simulink model "ofdm_txrx_supermimo"  (Simulink 7.1)
settings: Solver=VariableStepDiscrete  SolverMode=SingleTasking  StartTime=0.0  StopTime=simOnly_simLength  RelTol=1e-3
PostLoadFcn:
  | ofdm_rx_supermimo_init
InitFcn:
  | ofdm_rx_supermimo_init
StartFcn:
  | ofdm_rx_supermimo_init
values from neighbouring files (symbols this model uses):
  Action0_Config = 0* 31*2^18 + 0*2^24 + PHY_AUTORESPONSE_REQ_GOODHDR + PHY_AUTORESPONSE_REQ_GOODPKT + PHY_AUTORESPONSE_REQ_FLAGA    [ofdm_tx_supermimo_init.m]
  Action1_Config = 0* 62*2^18 + PHY_AUTORESPONSE_REQ_GOODHDR + PHY_AUTORESPONSE_REQ_GOODPKT    [ofdm_tx_supermimo_init.m]
  Action2_Config = 0    [ofdm_tx_supermimo_init.m]
  Action3_Config = 0    [ofdm_tx_supermimo_init.m]
  Action4_Config = 0    [ofdm_tx_supermimo_init.m]
  Action5_Config = 0    [ofdm_tx_supermimo_init.m]
  ActionID_AFTransmit = 31    [ofdm_tx_supermimo_init.m]
  ActionID_Disabled = 0    [ofdm_tx_supermimo_init.m]
  ActionID_SetFlagA = 62    [ofdm_tx_supermimo_init.m]
  ActionID_SetFlagB = 61    [ofdm_tx_supermimo_init.m]
  BER_RAM_init_size = 2048    [ofdm_tx_supermimo_init.m]
  CPLength = 16    [ofdm_tx_supermimo_init.m]
  longCorr_coef_i = [-4*real(LTS) 0]    [ofdm_rx_supermimo_init.m]
  longCorr_coef_q = [4*imag(LTS) 0]    [ofdm_rx_supermimo_init.m]
  Match0_Config = 0    [ofdm_tx_supermimo_init.m]
  Match1_Config = 0    [ofdm_tx_supermimo_init.m]
  Match2_Config = 0    [ofdm_tx_supermimo_init.m]
  Match3_Config = 0    [ofdm_tx_supermimo_init.m]
  Match4_Config = 0    [ofdm_tx_supermimo_init.m]
  Match5_Config = 0    [ofdm_tx_supermimo_init.m]
  Match6_Config = 0    [ofdm_tx_supermimo_init.m]
  Match7_Config = 0    [ofdm_tx_supermimo_init.m]
  max_CP_length = 16    [ofdm_tx_supermimo_init.m]
  max_num_baseRateSymbols = 31    [ofdm_tx_supermimo_init.m]
  max_num_subcarriers = 64    [ofdm_tx_supermimo_init.m]
  max_num_trainingSymbols = 15    [ofdm_tx_supermimo_init.m]
  max_numBytes = 16384    [ofdm_tx_supermimo_init.m]
  max_OFDM_symbols = 511    [ofdm_tx_supermimo_init.m]
  modConstellation_qam16 = .75*[1 3 -1 -3]./3    [ofdm_tx_supermimo_init.m]
  modConstellation_qam64 = 0.875*[3 1 5 7 -3 -1 -5 -7]./7    [ofdm_tx_supermimo_init.m]
  modConstellation_qpsk = [1 -1]./sqrt(2)    [ofdm_tx_supermimo_init.m]
  numBaseRateSymbols = ceil(numHeaderBytes / numBytes_BaseRateOFDMSymbol)    [ofdm_tx_supermimo_init.m]
  numSubcarriers = 64    [ofdm_tx_supermimo_init.m]
  numTrainingSymbols = 2    [ofdm_tx_supermimo_init.m]
  pilot_indicies = 7 + ( (64-21) * 2^8) + ( (64-7) * 2^16) + (21 * 2^24)    (= 356068103)    [ofdm_tx_supermimo_init.m]
  pilotValues = hex2dec('A57D') + (2^16 * hex2dec('5A82'))    [ofdm_tx_supermimo_init.m]
  pkt_crossCorr_thresh = 0.7    [ofdm_rx_supermimo_init.m]
  pkt_energy_thresh = 0    [ofdm_rx_supermimo_init.m]
  pktByteNums = numHeaderBytes +  				byteIndex_numPayloadBytes(1)*2^8 +  				byteIndex_numPayloadBytes(2)*2^16 +  				byteIndex_simpleDynModMasks * 2^24    [ofdm_rx_supermimo_init.m]
  PktDet_Delay = 58+32 +      Symbol_Timing_Offset*2^7 +      0*2^16 +      (117* 2^24)    (= 1962935642)    [ofdm_rx_supermimo_init.m]
  pktTiming_count_payloadStart = pktTiming_count_lsStart + pktTiming_ls_num*pktTiming_ls_length    [ofdm_rx_supermimo_init.m]
  preamble = preamble_scale*[shortsyms_10 longsyms_2]    [ofdm_tx_supermimo_init.m]
  preamble_ROM = preamble_I_ROM + preamble_Q_ROM*2^16    [ofdm_tx_supermimo_init.m]
  RAM_init_size = max_numBytes    (= 16384)    [ofdm_tx_supermimo_init.m]
  reg_AF_Tx_Blanking = (320+80) + ( (320+80+79)*2^16)    (= 31392144)    [ofdm_rx_supermimo_init.m]
  reg_AF_Tx_Scaling = 2944    [ofdm_rx_supermimo_init.m]
  reg_Interrupt_PktBuf_Control = reg_InterruptControl +  	1 * 2^16 +  	0 * 2^24    (= 65656)    [ofdm_rx_supermimo_init.m]
  reg_PilotCalcParams = (4 * 2^16) + (minPilotChanMag)    (= 262184.96)    [ofdm_rx_supermimo_init.m]
  regRx_chanEst_minMag = ((0.5)*2^15) + ((0.5 * 2^15)*2^16)    (= 1073758208)    [ofdm_rx_supermimo_init.m]
  regRx_coarseCalcCorrection = round((1.3e-3 - 1.2982e-3)*2^32)    [ofdm_rx_supermimo_init.m]
  regRx_pilotCalcCorrection = round(1.00115 * 2^31)    [ofdm_rx_supermimo_init.m]
  regRx_preCFOoptions = 0 * 2^0 +  	1 * 2^1 +  	0    (= 2)    [ofdm_rx_supermimo_init.m]
  rx_controlBits = 1 * 2  		 + 1 * 4  		 + 0 * 8  		 + 1 * 16 * rx_SISO_Mode  		 + 1 * 32  		 + 1 * 64  		 + 1 * 128  		 + 1 * 256  		 + 1 * 512  		 + 1 * 1024  		 + 1 * 4096  		 + 0 * 16384  		 + 0 * 32768  		 + 1 * 65536  		 + 0 * 131072  		 + 0 * 262144  		 + 1 * 524288  		 + 1 * 1048576 * tx_Alamouti_Mode  		 + 0 * 2097152           + 1 * 33554432  		 + 0 * 4194304  		 + 0 * 8388608  		 + 0 * 16777216  		 + 1 *  ...(+49 chars)    [ofdm_rx_supermimo_init.m]
  Rx_PktDet_LongCorr_Params = LongCorrCounterLoad + 0*2^8 + 0*2^16 + 255*2^24    (= 4278190325)    [ofdm_rx_supermimo_init.m]
  Rx_PktDet_LongCorrThresholds = 8000    [ofdm_rx_supermimo_init.m]
  rx_postEq_scaling = round(rxScaling*2^11) * (1 + 2^16)    [ofdm_rx_supermimo_init.m]
  RxDataScrambling_Seq = [40 198 78 63 82 173 102 245 48 111 172 115 147 230 216 93 72 65 62 2 205 242 122 90 128 83 105 97 73 10 5 252]    [ofdm_tx_supermimo_init.m]
  RxReg_FixedPktLen = 0    [ofdm_rx_supermimo_init.m]
  shortSymbol_time = shortSymbol_time(1:16).'    [ofdm_tx_supermimo_init.m]
  simOnly_numSamples = length(preamble)+( (numSubcarriers+CPLength)*(numTrainingSymbols + numBaseRateSymbols + numFullRateSymbols/2) )    [ofdm_tx_supermimo_init.m]
  train = [train train]    [ofdm_tx_supermimo_init.m]
  tx_controlBits = (antB_preambleShift * 2^4) + (2^1 * tx_Alamouti_Mode) +  	(2^0 * tx_SISO_Mode) + 4*0 + 8*1 + 0*256 + 512*0 + 1024*0 +  	0*hex2dec('1000') + 0*hex2dec('2000') + 0*hex2dec('4000') +  	1*hex2dec('8000') + 1*hex2dec('10000') + hex2dec('20000') +  	16*hex2dec('100000')    [ofdm_tx_supermimo_init.m]
  tx_delays = (50*2^0) + (0*2^8) + (30*2^12)    (= 122930)    [ofdm_tx_supermimo_init.m]
  tx_scaling = (3072) + (12288 * 2^16)    (= 805309440)    [ofdm_tx_supermimo_init.m]
  TxDataScrambling_Seq = [40 198 78 63 82 173 102 245 48 111 172 115 147 230 216 93 72 65 62 2 205 242 122 90 128 83 105 97 73 10 5 252]    [ofdm_tx_supermimo_init.m]
  txfilt_firpm = 0.95*txfilt_firpm./(max(txfilt_firpm))    [ofdm_tx_supermimo_init.m]
  txfilt_intfilt = [0 intfilt(4,4,.8)]    [ofdm_tx_supermimo_init.m]
  txReg_symbolCounts = (2^8 *numBaseRateSymbols ) + numTrainingSymbols    [ofdm_tx_supermimo_init.m]
  TxRx_FFTScaling = (3 * 2^0) +  	(2 * 2^2) +  	(1 * 2^4) +  	(1 * 2^6) +  	(1 * 2^8) +  	(0 * 2^10)    (= 347)    [ofdm_tx_supermimo_init.m]
undefined symbols (not defined in the file or in its folder): AntA, AntB, BaseRate, Down, gcbh, numBuffs, numBytes, Up, xlBlockLoadCallback, xlGetSimulinkPeriod, xlipgetpartsetting
libraries: xbsIndex_r4 x6035, simulink x4, dspsigops x4, commchan3 x2, dspsrcs4 x2, commrflib2 x2
(part 19 of 61)

== system ofdm_txrx_supermimo/OFDM Rx MIMO/Equalizer & Packetizer/Equalizer/Determinant ==
  note: In SISO and Multiplexing mode, this block
      | calculates the determinant of the 2x2 matrix:
      | det([a b; c d]) = ad - bc
      | where a,b,c,d are all complex
      | In Alamouti mode, it calculates the sum of
      | the magnitude of the two channel coefficients
      | |a|^2 + |b|^2
  Re[a] : Inport  OutDataType=sfix(16)  OutScaling=2^0
  Im[a] : Inport  Port=2  OutDataType=sfix(16)  OutScaling=2^0
  Re[d] : Inport  Port=3  OutDataType=sfix(16)  OutScaling=2^0
  Im[d] : Inport  Port=4  OutDataType=sfix(16)  OutScaling=2^0
  Re[b] : Inport  Port=5  OutDataType=sfix(16)  OutScaling=2^0
  Im[b] : Inport  Port=6  OutDataType=sfix(16)  OutScaling=2^0
  Re[c] : Inport  Port=7  OutDataType=sfix(16)  OutScaling=2^0
  Im[c] : Inport  Port=8  OutDataType=sfix(16)  OutScaling=2^0
  Add1 : xbsIndex_r4/AddSub  mode=Addition or Subtraction  use_carryin=off  use_carryout=off  en=off  latency=0  precision=User Defined  arith_type="Signed  (2's comp)"  n_bits=22  bin_pt=17  quantization=Truncate  overflow=Flag as error  dbl_ovrd=off  use_behavioral_HDL=on  pipelined=off  use_rpm=on  hw_selection=Fabric  block_type=addsub
      settings: xl_area=[9 0 0 16 0 0 0]  block_version=VER_STRING_GOES_HERE
  Add2 : xbsIndex_r4/AddSub  mode=Addition or Subtraction  use_carryin=off  use_carryout=off  en=off  latency=0  precision=User Defined  arith_type="Signed  (2's comp)"  n_bits=22  bin_pt=17  quantization=Truncate  overflow=Flag as error  dbl_ovrd=off  use_behavioral_HDL=on  pipelined=off  use_rpm=on  hw_selection=Fabric  block_type=addsub
      settings: xl_area=[9 0 0 16 0 0 0]  block_version=VER_STRING_GOES_HERE
  ComplexMult_x1 : Subsystem
  ComplexMult_x2 : Subsystem
  Inverter : xbsIndex_r4/Inverter  en=off  latency=0  dbl_ovrd=off  block_type=inv
  Re : Outport  OutDataType=sfix(16)  OutScaling=2^0
  Im : Outport  Port=2  OutDataType=sfix(16)  OutScaling=2^0
  wires:
  ComplexMult_x2/1(Re) -> Add2/1
  ComplexMult_x1/1(Re) -> Add2/2
  ComplexMult_x2/2(Im) -> Add1/1
  ComplexMult_x1/2(Im) -> Add1/2
  Re[a]/1 -> ComplexMult_x2/1(Re[A])
  Im[a]/1 -> ComplexMult_x2/2(Im[A])
  Add2/1 -> Re/1
  Re[b]/1 -> ComplexMult_x1/1(Re[A])
  Add1/1 -> Im/1
  Im[b]/1 -> ComplexMult_x1/2(Im[A])
  [regRx_AlamoutiMode] -> Inverter/1
  Inverter/1 -> Add2/3, Add1/3
  Re[c]/1 -> ComplexMult_x1/3(Re[B])
  Im[c]/1 -> ComplexMult_x1/4(Im[B])
  Re[d]/1 -> ComplexMult_x2/3(Re[B])
  Im[d]/1 -> ComplexMult_x2/4(Im[B])

== system ofdm_txrx_supermimo/OFDM Rx MIMO/Equalizer & Packetizer/Equalizer/Phase Corr ==
  note: For SISO/Multiplexing, update the phase correction
      | value for each new symbol.
      | For Alamouti, update it every other symbol
      | (since in Alamouti mode the sym index increments
      | twice for each pair of coincident symbols).
  Vin : Inport  OutDataType=sfix(16)  OutScaling=2^0
  xk_ind : Inport  Port=2  OutDataType=sfix(16)  OutScaling=2^0
  Phases I : Inport  Port=3  OutDataType=sfix(16)  OutScaling=2^0
  Phases Q : Inport  Port=4  OutDataType=sfix(16)  OutScaling=2^0
  YI_A : Inport  Port=5
  YQ_A : Inport  Port=6
  YI_B : Inport  Port=7
  YQ_B : Inport  Port=8
  Bus Selector1 : BusSelector  OutputSignals=signal1,signal2
  Bus Selector2 : BusSelector  OutputSignals=signal1,signal2
  ComplexMult_x3 : Subsystem
  ComplexMult_x4 : Subsystem
  Phase Corr : Scope  NumInputPorts=7
      settings: TimeRange=392.7801724137931  YMin=-0.5~-0.25~-1~-1~0~0~0  YMax=0.5~0.15~1~1~1~3500~1  SaveName=ScopeData21
  done{1..7} (7 alike) : xbsIndex_r4/Gateway Out  hdl_port=off  timing_constraint=None  locs_specified=off  LOCs={}  block_type=gatewayout
      settings: infoedit="Gateway out block.  Converts Xilinx fixed point inputs into ouputs of type Simulink integer, double, or fixed point.<P><P>Hardware notes:  In hardware these blocks become top level output ports or are discarded, depending on how they are configured."  xl_area=[0 0 0 0 1 0 0]  block_version=VER_STRING_GOES_HERE
  " YI_A" : Outport
  " YQ_A" : Outport  Port=2
  " YI_B" : Outport  Port=3
  " YQ_B" : Outport  Port=4
  wires:
  ComplexMult_x4/1(Re) -> " YI_B"/1
  ComplexMult_x4/2(Im) -> " YQ_B"/1
  YI_B/1 -> ComplexMult_x4/1(Re[A]), done7/1
  YQ_B/1 -> ComplexMult_x4/2(Im[A])
  YI_A/1 -> ComplexMult_x3/1(Re[A]), done6/1
  YQ_A/1 -> ComplexMult_x3/2(Im[A])
  ComplexMult_x3/1(Re) -> " YI_A"/1
  ComplexMult_x3/2(Im) -> " YQ_A"/1
  Phases I/1 -> Bus Selector1/1
  Bus Selector1/1 -> ComplexMult_x3/3(Re[B]), done5/1  "<signal1>"
  Bus Selector1/2 -> ComplexMult_x4/3(Re[B]), done2/1  "<signal2>"
  Phases Q/1 -> Bus Selector2/1
  Bus Selector2/1 -> ComplexMult_x3/4(Im[B]), done1/1  "<signal1>"
  Bus Selector2/2 -> ComplexMult_x4/4(Im[B]), done3/1  "<signal2>"
  done4/1 -> Phase Corr/1
  done5/1 -> Phase Corr/2
  xk_ind/1 -> done4/1
  done1/1 -> Phase Corr/3
  done2/1 -> Phase Corr/4
  done3/1 -> Phase Corr/5
  done6/1 -> Phase Corr/6
  done7/1 -> Phase Corr/7

== system ofdm_txrx_supermimo/OFDM Rx MIMO/Equalizer & Packetizer/Equalizer/YA*[HBB,-HAB] ==
  Re[y] : Inport  OutDataType=sfix(16)  OutScaling=2^0
  Im[y] : Inport  Port=2  OutDataType=sfix(16)  OutScaling=2^0
  Re[h1] : Inport  Port=3  OutDataType=sfix(16)  OutScaling=2^0
  Im[h1] : Inport  Port=4  OutDataType=sfix(16)  OutScaling=2^0
  Re[h2] : Inport  Port=5  OutDataType=sfix(16)  OutScaling=2^0
  Im[h2] : Inport  Port=6  OutDataType=sfix(16)  OutScaling=2^0
  Slot : Inport  Port=7  OutDataType=sfix(16)  OutScaling=2^0
  ComplexMult_x1 : Subsystem
  Concat : xbsIndex_r4/Concat  num_inputs=2  dbl_ovrd=off  block_type=concat
      settings: block_version=10.1.3
  Mux1 : xbsIndex_r4/Mux  inputs=4  en=off  latency=0  precision=Full  arith_type="Signed  (2's comp)"  n_bits=16  bin_pt=13  quantization=Truncate  overflow=Wrap  dbl_ovrd=off  block_type=mux
      settings: xl_area=[14 0 0 28 0 0 0]  block_version=VER_STRING_GOES_HERE
  Mux2 : xbsIndex_r4/Mux  inputs=4  en=off  latency=0  precision=Full  arith_type="Signed  (2's comp)"  n_bits=16  bin_pt=13  quantization=Truncate  overflow=Wrap  dbl_ovrd=off  block_type=mux
      settings: xl_area=[14 0 0 28 0 0 0]  block_version=VER_STRING_GOES_HERE
  Negate1 : xbsIndex_r4/Negate  precision=User Defined  arith_type="Signed  (2's comp)"  n_bits=18  bin_pt=16  quantization=Truncate  overflow=Flag as error  en=off  latency=0  dbl_ovrd=off  block_type=negate
      settings: xl_area=[7 0 0 14 0 0 0]  block_version=VER_STRING_GOES_HERE
  Negate2 : xbsIndex_r4/Negate  precision=User Defined  arith_type="Signed  (2's comp)"  n_bits=18  bin_pt=16  quantization=Truncate  overflow=Flag as error  en=off  latency=0  dbl_ovrd=off  block_type=negate
      settings: xl_area=[7 0 0 14 0 0 0]  block_version=VER_STRING_GOES_HERE
  Re : Outport  OutDataType=sfix(16)  OutScaling=2^0
  Im : Outport  Port=2  OutDataType=sfix(16)  OutScaling=2^0
  wires:
  Re[y]/1 -> ComplexMult_x1/1(Re[A])
  ComplexMult_x1/1(Re) -> Re/1
  Im[y]/1 -> ComplexMult_x1/2(Im[A])
  Re[h1]/1 -> Mux2/4, Mux2/2
  ComplexMult_x1/2(Im) -> Im/1
  Re[h2]/1 -> Negate2/1, Mux2/5
  Im[h2]/1 -> Negate1/1, Mux1/5
  Negate2/1 -> Mux2/3
  [regRx_AlamoutiMode] -> Concat/1
  Slot/1 -> Concat/2
  Concat/1 -> Mux1/1, Mux2/1
  Im[h1]/1 -> Mux1/2, Mux1/4
  Mux1/1 -> ComplexMult_x1/4(Im[B])
  Mux2/1 -> ComplexMult_x1/3(Re[B])
  Negate1/1 -> Mux1/3

== system ofdm_txrx_supermimo/OFDM Rx MIMO/Equalizer & Packetizer/Equalizer/YB*[-HBA,HAA] ==
  Re[y] : Inport  OutDataType=sfix(16)  OutScaling=2^0
  Im[y] : Inport  Port=2  OutDataType=sfix(16)  OutScaling=2^0
  Re[h1] : Inport  Port=3  OutDataType=sfix(16)  OutScaling=2^0
  Im[h1] : Inport  Port=4  OutDataType=sfix(16)  OutScaling=2^0
  Re[h2] : Inport  Port=5  OutDataType=sfix(16)  OutScaling=2^0
  Im[h2] : Inport  Port=6  OutDataType=sfix(16)  OutScaling=2^0
  Slot : Inport  Port=7  OutDataType=sfix(16)  OutScaling=2^0
  ComplexMult_x2 : Subsystem
  Concat : xbsIndex_r4/Concat  num_inputs=2  dbl_ovrd=off  block_type=concat
      settings: block_version=10.1.3
  Mux1 : xbsIndex_r4/Mux  inputs=2  en=off  latency=0  precision=Full  arith_type="Signed  (2's comp)"  n_bits=16  bin_pt=13  quantization=Truncate  overflow=Wrap  dbl_ovrd=off  block_type=mux
      settings: xl_area=[14 0 0 28 0 0 0]  block_version=VER_STRING_GOES_HERE
  Mux2 : xbsIndex_r4/Mux  inputs=4  en=off  latency=0  precision=Full  arith_type="Signed  (2's comp)"  n_bits=16  bin_pt=13  quantization=Truncate  overflow=Wrap  dbl_ovrd=off  block_type=mux
      settings: xl_area=[14 0 0 28 0 0 0]  block_version=VER_STRING_GOES_HERE
  Mux3 : xbsIndex_r4/Mux  inputs=4  en=off  latency=0  precision=Full  arith_type="Signed  (2's comp)"  n_bits=16  bin_pt=13  quantization=Truncate  overflow=Wrap  dbl_ovrd=off  block_type=mux
      settings: xl_area=[14 0 0 28 0 0 0]  block_version=VER_STRING_GOES_HERE
  Negate{1..5} (5 alike) : xbsIndex_r4/Negate  precision=User Defined  arith_type="Signed  (2's comp)"  n_bits=18  bin_pt=16  quantization=Truncate  overflow=Flag as error  en=off  latency=0  dbl_ovrd=off  block_type=negate
      settings: xl_area=[7 0 0 14 0 0 0]  block_version=VER_STRING_GOES_HERE
  Re : Outport  OutDataType=sfix(16)  OutScaling=2^0
  Im : Outport  Port=2  OutDataType=sfix(16)  OutScaling=2^0
  wires:
  Im[h1]/1 -> Negate2/1, Mux2/4
  ComplexMult_x2/2(Im) -> Im/1
  Re[h1]/1 -> Negate1/1, Mux3/4
  ComplexMult_x2/1(Re) -> Re/1
  [regRx_AlamoutiMode] -> Concat/1, Mux1/1
  Slot/1 -> Concat/2
  Concat/1 -> Mux3/1, Mux2/1
  Negate1/1 -> Mux3/2
  Negate2/1 -> Mux2/2
  Re[h2]/1 -> Mux3/3, Negate3/1
  Im[h2]/1 -> Mux2/3, Negate4/1
  Negate3/1 -> Mux3/5
  Negate4/1 -> Mux2/5
  Re[y]/1 -> ComplexMult_x2/1(Re[A])
  Mux1/1 -> ComplexMult_x2/2(Im[A])
  Im[y]/1 -> Mux1/2, Negate5/1
  Negate5/1 -> Mux1/3
  Mux3/1 -> ComplexMult_x2/3(Re[B])
  Mux2/1 -> ComplexMult_x2/4(Im[B])

== system ofdm_txrx_supermimo/OFDM Rx MIMO/Equalizer & Packetizer/Packet_Constructor/   ==
  signal : Inport  OutDataType=sfix(16)  OutScaling=2^0
  Delay1 : xbsIndex_r4/Delay  en=off  latency=1  dbl_ovrd=off  reg_retiming=off  block_type=delay
      settings: infoedit=Hardware notes: A delay line is a chain, each link of which is an SRL16 followed by a flip-flop. If register retiming is enabled, the delay line is a chain of flip-flops.  xl_area=[1 1 0 0 0 0 0]  block_version=VER_STRING_GOES_HERE
  Inverter1 : xbsIndex_r4/Inverter  en=off  latency=0  dbl_ovrd=off  block_type=inv
      settings: xl_area=[0 0 0 0 0 0 0]  block_version=VER_STRING_GOES_HERE
  Logical1 : xbsIndex_r4/Logical  logical_function=AND  inputs=2  en=off  latency=0  precision=Full  arith_type=Unsigned  n_bits=8  bin_pt=2  align_bp=on  dbl_ovrd=off  block_type=logical
      settings: xl_area=[1 0 0 1 0 0 0]  block_version=VER_STRING_GOES_HERE
  pulse : Outport  OutDataType=sfix(16)  OutScaling=2^0
  wires:
  Inverter1/1 -> Delay1/1
  signal/1 -> Inverter1/1, Logical1/1
  Delay1/1 -> Logical1/2
  Logical1/1 -> pulse/1

== system ofdm_txrx_supermimo/OFDM Rx MIMO/Equalizer & Packetizer/Packet_Constructor/Barrel Shifter ==
  ShiftSel : Inport  OutDataType=sfix(16)  OutScaling=2^0
  D : Inport  Port=2  OutDataType=sfix(16)  OutScaling=2^0
  en : Inport  Port=3  OutDataType=sfix(16)  OutScaling=2^0
  AddSub1 : xbsIndex_r4/AddSub  mode=Addition  use_carryin=off  use_carryout=off  en=off  latency=0  precision=User Defined  arith_type=Unsigned  n_bits=15  bin_pt=0  quantization=Truncate  overflow=Wrap  dbl_ovrd=off  use_behavioral_HDL=on  pipelined=off  use_rpm=on  hw_selection=Fabric  block_type=addsub
      settings: xl_area=[8 0 0 15 0 0 0]  block_version=VER_STRING_GOES_HERE
  Assert2 : xbsIndex_r4/Assert  Orientation=down  assert_type=off  type_source=Explicitly  arith_type="Signed  (2's comp)"  n_bits=16  bin_pt=14  assert_rate=on  rate_source=Explicitly  period=2  output_port=on  block_type=assert
      settings: infoedit=Asserts a user-defined sample rate and/or type on a signal.<P><P>Hardware notes: In hardware this block costs nothing.  block_version=VER_STRING_GOES_HERE
  Data Buffer : xbsIndex_r4/Register  Orientation=left  init=0  rst=off  en=on  dbl_ovrd=off  block_type=register
      settings: xl_area=[8 15 0 0 0 0 0]  block_version=VER_STRING_GOES_HERE
  Delay2 : xbsIndex_r4/Delay  en=off  latency=1  dbl_ovrd=off  reg_retiming=off  block_type=delay
      settings: infoedit=Hardware notes: A delay line is a chain, each link of which is an SRL16 followed by a flip-flop. If register retiming is enabled, the delay line is a chain of flip-flops.  xl_area=[1 1 0 0 0 0 0]  block_version=VER_STRING_GOES_HERE
  Shift : xbsIndex_r4/Shift  shift_dir=Left  shift_bits=1  en=off  latency=0  precision=User Defined  arith_type=Unsigned  n_bits=15  bin_pt=0  quantization=Truncate  overflow=Wrap  dbl_ovrd=off  block_type=shift
      settings: xl_area=[0 0 0 0 0 0 0]  block_version=VER_STRING_GOES_HERE
  Shift1 : xbsIndex_r4/Shift  shift_dir=Left  shift_bits=2  en=off  latency=0  precision=User Defined  arith_type=Unsigned  n_bits=15  bin_pt=0  quantization=Truncate  overflow=Wrap  dbl_ovrd=off  block_type=shift
      settings: xl_area=[0 0 0 0 0 0 0]  block_version=VER_STRING_GOES_HERE
  Shift2 : xbsIndex_r4/Shift  shift_dir=Left  shift_bits=4  en=off  latency=0  precision=User Defined  arith_type=Unsigned  n_bits=15  bin_pt=0  quantization=Truncate  overflow=Wrap  dbl_ovrd=off  block_type=shift
      settings: xl_area=[0 0 0 0 0 0 0]  block_version=VER_STRING_GOES_HERE
  Shift3 : xbsIndex_r4/Shift  shift_dir=Left  shift_bits=6  en=off  latency=0  precision=User Defined  arith_type=Unsigned  n_bits=15  bin_pt=0  quantization=Truncate  overflow=Wrap  dbl_ovrd=off  block_type=shift
      settings: xl_area=[0 0 0 0 0 0 0]  block_version=VER_STRING_GOES_HERE
  Shift4 : xbsIndex_r4/Shift  shift_dir=Left  shift_bits=8  en=off  latency=0  precision=User Defined  arith_type=Unsigned  n_bits=15  bin_pt=0  quantization=Truncate  overflow=Wrap  dbl_ovrd=off  block_type=shift
      settings: xl_area=[0 0 0 0 0 0 0]  block_version=VER_STRING_GOES_HERE
  Shift5 : xbsIndex_r4/Shift  shift_dir=Left  shift_bits=3  en=off  latency=0  precision=User Defined  arith_type=Unsigned  n_bits=15  bin_pt=0  quantization=Truncate  overflow=Wrap  dbl_ovrd=off  block_type=shift
      settings: xl_area=[0 0 0 0 0 0 0]  block_version=VER_STRING_GOES_HERE
  Shift6 : xbsIndex_r4/Shift  shift_dir=Left  shift_bits=5  en=off  latency=0  precision=User Defined  arith_type=Unsigned  n_bits=15  bin_pt=0  quantization=Truncate  overflow=Wrap  dbl_ovrd=off  block_type=shift
      settings: xl_area=[0 0 0 0 0 0 0]  block_version=VER_STRING_GOES_HERE
  Shift7 : xbsIndex_r4/Shift  shift_dir=Left  shift_bits=7  en=off  latency=0  precision=User Defined  arith_type=Unsigned  n_bits=15  bin_pt=0  quantization=Truncate  overflow=Wrap  dbl_ovrd=off  block_type=shift
      settings: xl_area=[0 0 0 0 0 0 0]  block_version=VER_STRING_GOES_HERE
  shift selector : xbsIndex_r4/Mux  inputs=9  en=off  latency=0  precision=Full  arith_type="Signed  (2's comp)"  n_bits=8  bin_pt=2  quantization=Truncate  overflow=Wrap  dbl_ovrd=off  block_type=mux
      settings: xl_area=[68 0 0 135 0 0 0]  block_version=VER_STRING_GOES_HERE
  Q : Outport  OutDataType=sfix(16)  OutScaling=2^0
  wires:
  D/1 -> Delay2/1
  ShiftSel/1 -> shift selector/1
  Shift7/1 -> shift selector/9
  Shift6/1 -> shift selector/7
  Shift5/1 -> shift selector/5
  shift selector/1 -> AddSub1/2
  Shift4/1 -> shift selector/10
  Shift3/1 -> shift selector/8
  Shift2/1 -> shift selector/6
  Shift1/1 -> shift selector/4
  Shift/1 -> shift selector/3
  AddSub1/1 -> Assert2/1
  Data Buffer/1 -> Shift5/1, shift selector/2, Shift/1, Shift1/1, Shift2/1, Shift6/1, Shift3/1, Shift7/1, Shift4/1
  en/1 -> Data Buffer/2
  Delay2/1 -> AddSub1/1
  Assert2/1 -> Q/1, Data Buffer/1

== system ofdm_txrx_supermimo/OFDM Rx MIMO/Equalizer & Packetizer/Packet_Constructor/Data Buffer ==
  Payload_Addr : Inport  OutDataType=sfix(16)  OutScaling=2^0
  Payload_WE : Inport  Port=2  OutDataType=sfix(16)  OutScaling=2^0
  Payload_Data : Inport  Port=3  OutDataType=sfix(16)  OutScaling=2^0
  Rst : Inport  Port=4  OutDataType=sfix(16)  OutScaling=2^0
  PktDone : Inport  Port=5  OutDataType=sfix(16)  OutScaling=2^0
  AutoResponse Processing : Subsystem
  BER Calc : Subsystem
  Descrambling & Pkt Buffer : Subsystem
  Error Checking : Subsystem
  Header Decoding : Subsystem
  numBytes : Outport  OutDataType=sfix(16)  OutScaling=2^0
  modMask : Outport  Port=2  OutDataType=sfix(16)  OutScaling=2^0
  ResetRx : Outport  Port=3  OutDataType=sfix(16)  OutScaling=2^0
  wires:
  Payload_Addr/1 -> Descrambling & Pkt Buffer/1(Addr)
  Payload_Data/1 -> Descrambling & Pkt Buffer/2(Data)
  Payload_WE/1 -> Descrambling & Pkt Buffer/3(We)
  Rst/1 -> Error Checking/5(Rst), AutoResponse Processing/1(Rst), Header Decoding/4(Rst), BER Calc/4(PktStart)
  Descrambling & Pkt Buffer/1(" Addr") -> Header Decoding/1(RxByteAddr), Error Checking/2(ByteAddr), AutoResponse Processing/2(RxByteAddr), BER Calc/1(BER_addr)
  Descrambling & Pkt Buffer/2(" Data") -> Header Decoding/2(RxByte), Error Checking/3(ByteIn), AutoResponse Processing/3(RxByte), BER Calc/2(BER_data)
  Descrambling & Pkt Buffer/3(" Vout") -> Header Decoding/3(RxByteWE), Error Checking/4(Vin), AutoResponse Processing/4(RxByteWE), BER Calc/3(BER_val)
  Header Decoding/1(numBytes) -> Error Checking/1(NumBytes), BER Calc/5(numBytes), numBytes/1
  Header Decoding/2(modMask) -> modMask/1
  PktDone/1 -> AutoResponse Processing/5(PktDone)
  Error Checking/1(ResetRx) -> ResetRx/1

== system ofdm_txrx_supermimo/OFDM Rx MIMO/Equalizer & Packetizer/Packet_Constructor/EVM Calc ==
  Rx Bits : Inport
  Mod Sel : Inport  Port=2
  Eq I : Inport  Port=3
  Eq Q : Inport  Port=4
  Xk_ind : Inport  Port=5
  Sym_ind : Inport  Port=6
  Vin : Inport  Port=7
  Done : Inport  Port=8
  AddSub : xbsIndex_r4/AddSub  mode=Subtraction  use_carryin=off  use_carryout=off  en=off  latency=0  precision=User Defined  arith_type="Signed  (2's comp)"  n_bits=16  bin_pt=13  quantization=Truncate  overflow=Flag as error  dbl_ovrd=off  use_behavioral_HDL=off  pipelined=off  use_rpm=on  hw_selection=Fabric  block_type=addsub
      settings: block_version=10.1.3
  AddSub1 : xbsIndex_r4/AddSub  mode=Subtraction  use_carryin=off  use_carryout=off  en=off  latency=0  precision=User Defined  arith_type="Signed  (2's comp)"  n_bits=16  bin_pt=13  quantization=Truncate  overflow=Flag as error  dbl_ovrd=off  use_behavioral_HDL=off  pipelined=off  use_rpm=on  hw_selection=Fabric  block_type=addsub
      settings: block_version=10.1.3
  ComplexMag (Latency=2) : Subsystem
  Constant : xbsIndex_r4/Constant  arith_type=Unsigned  const=0  n_bits=1  bin_pt=0  explicit_period=off  period=1  equ=P=C  opselect=C  inp2=PCIN>>17  opr=+  inp1=P  carry=CIN  dbl_ovrd=off  block_type=constant
  Convert1 : xbsIndex_r4/Convert  arith_type="Signed  (2's comp)"  n_bits=14  bin_pt=13  quantization=Truncate  overflow=Saturate  latency=0  dbl_ovrd=off  pipeline=off  block_type=convert
      settings: xl_area=[0, 0, 0, 0, 0, 0, 0]  block_version=VER_STRING_GOES_HERE
  Delay1 : xbsIndex_r4/Delay  en=off  latency=2  dbl_ovrd=off  reg_retiming=off  block_type=delay
  Delay2 : xbsIndex_r4/Delay  en=off  latency=4  dbl_ovrd=off  reg_retiming=off  block_type=delay
  Delay3 : xbsIndex_r4/Delay  en=off  latency=2  dbl_ovrd=off  reg_retiming=off  block_type=delay
  Delay4 : xbsIndex_r4/Delay  en=off  latency=2  dbl_ovrd=off  reg_retiming=off  block_type=delay
  Delay5 : xbsIndex_r4/Delay  en=off  latency=4  dbl_ovrd=off  reg_retiming=off  block_type=delay
  Delay6 : xbsIndex_r4/Delay  en=off  latency=4  dbl_ovrd=off  reg_retiming=off  block_type=delay
  Delay7 : xbsIndex_r4/Delay  en=off  latency=1  dbl_ovrd=off  reg_retiming=off  block_type=delay
  Delay8 : xbsIndex_r4/Delay  en=off  latency=1  dbl_ovrd=off  reg_retiming=off  block_type=delay
  "EVM " : Scope  NumInputPorts=7
      settings: TimeRange=8000  YMin=0~0~-0.75~-0.75~0~0~0  YMax=70~12.5~0.75~0.75~1~1~1  SaveName=ScopeData78
  EVM per Subcarrier : Subsystem
  EVM per Symbol : Subsystem
  Inverter : xbsIndex_r4/Inverter  en=off  latency=0  dbl_ovrd=off  block_type=inv
  Logical : xbsIndex_r4/Logical  logical_function=OR  inputs=3  en=off  latency=0  precision=Full  arith_type=Unsigned  n_bits=16  bin_pt=0  align_bp=on  dbl_ovrd=off  block_type=logical
  Posedge6 : Subsystem
  Re-modulator : Subsystem
  Register2 : xbsIndex_r4/Register  init=0  rst=on  en=off  dbl_ovrd=off  block_type=register
  Relational : xbsIndex_r4/Relational  mode=a=b  en=off  latency=1  dbl_ovrd=off  block_type=relational
      settings: block_version=10.1.3
  Rx_debug_EVM : xbsIndex_r4/Gateway Out  hdl_port=on  timing_constraint=None  locs_specified=off  LOCs={}  block_type=gatewayout
      settings: infoedit="Gateway out block.  Converts Xilinx fixed point inputs into ouputs of type Simulink integer, double, or fixed point.<P><P>Hardware notes:  In hardware these blocks become top level output ports or are discarded, depending on how they are configured."  xl_area=[0 0 0 0 1 0 0]  block_version=VER_STRING_GOES_HERE
  SRLatch : Subsystem
  Up Sample : xbsIndex_r4/Up Sample  sample_ratio=2  copy_samples=on  dbl_ovrd=off  block_type=usamp
      settings: infoedit="Up samples input data.  Inserted values  can be zeros or copies of the most recent input sample.<P><P>Hardware notes: No hardware is needed if inserted values are copies of the input sample; otherwise, a mux and single bit flip-flop are used."  block_version=8.2.02
  done{1..7} (7 alike) : xbsIndex_r4/Gateway Out  hdl_port=off  timing_constraint=None  locs_specified=off  LOCs={}  block_type=gatewayout
      settings: infoedit="Gateway out block.  Converts Xilinx fixed point inputs into ouputs of type Simulink integer, double, or fixed point.<P><P>Hardware notes:  In hardware these blocks become top level output ports or are discarded, depending on how they are configured."  xl_area=[0 0 0 0 1 0 0]  block_version=VER_STRING_GOES_HERE
  tag scope: DebugOut_EVM=global
  wires:
  Mod Sel/1 -> Re-modulator/2(Mod Sel), Relational/1
  Eq I/1 -> Delay4/1
  Re-modulator/1(I) -> AddSub/2
  Re-modulator/2(Q) -> AddSub1/2
  Eq Q/1 -> Delay3/1
  AddSub/1 -> ComplexMag (Latency=2)/1(Re[A])
  AddSub1/1 -> ComplexMag (Latency=2)/2(Im[A])
  ComplexMag (Latency=2)/1(abs(A)) -> done5/1, Register2/1
  Delay3/1 -> AddSub1/1, done3/1
  Constant/1 -> Relational/2
  Register2/1 -> done7/1, Convert1/1, [DebugOut_EVM], EVM per Subcarrier/1(EVM_d1), EVM per Symbol/1(EVM)
  Rx Bits/1 -> Re-modulator/1(Mod Bits)
  Relational/1 -> Delay1/1
  Delay4/1 -> AddSub/1, done1/1
  Delay1/1 -> Logical/1
  Vin/1 -> Delay2/1, Posedge6/1(D)
  Inverter/1 -> Logical/2
  Logical/1 -> Register2/2, done6/1, EVM per Subcarrier/2(Vin_d1#)
  Delay2/1 -> Inverter/1
  Up Sample/1 -> Rx_debug_EVM/1
  Convert1/1 -> Up Sample/1
  Xk_ind/1 -> Delay5/1
  done1/1 -> "EVM "/3  "Eq I"
  done3/1 -> "EVM "/4  "Eq Q"
  done5/1 -> "EVM "/5  "Raw EVM"
  done6/1 -> "EVM "/6  "Rst"
  done7/1 -> "EVM "/7  "Valid EVM"
  Delay5/1 -> EVM per Subcarrier/3(SC ind_d0), Delay8/1, done2/1
  done2/1 -> "EVM "/1  "Xk ind"
  done4/1 -> "EVM "/2  "Sym Ind"
  Sym_ind/1 -> Delay6/1
  Delay6/1 -> Delay7/1, EVM per Subcarrier/4(Sym Ind), done4/1
  Delay7/1 -> EVM per Symbol/3(Sym Ind)
  Delay8/1 -> EVM per Symbol/2(SC ind)
  SRLatch/1(Q) -> Logical/3
  Posedge6/1(Q) -> SRLatch/1(R)
  Done/1 -> SRLatch/2(S)

== system ofdm_txrx_supermimo/OFDM Rx MIMO/Equalizer & Packetizer/Packet_Constructor/Hard Decision ==
  note: This block demaps the signal according to its modulation scheme by slicing off a certain number of most signicant bits and then using that sliced number for a lookup table that
      | reverses the grey coding and produces the bit values.
  note: [0 1 3 2]
  Mod Sel : Inport  OutDataType=sfix(16)  OutScaling=2^0
  I : Inport  Port=2  OutDataType=sfix(16)  OutScaling=2^0
  Q : Inport  Port=3  OutDataType=sfix(16)  OutScaling=2^0
  Concat{,1,5..6} (4 alike) : xbsIndex_r4/Concat  num_inputs=2  dbl_ovrd=off  block_type=concat
      settings: block_version=VER_STRING_GOES_HERE
  Constant7 : xbsIndex_r4/Constant  arith_type=Unsigned  const=0  n_bits=8  bin_pt=0  explicit_period=off  period=1  equ=P=C  opselect=C  inp2=PCIN>>17  opr=+  inp1=P  carry=CIN  dbl_ovrd=off  block_type=constant
      settings: block_version=VER_STRING_GOES_HERE
  Delay : xbsIndex_r4/Delay  en=off  latency=1  dbl_ovrd=off  reg_retiming=off  block_type=delay
      settings: infoedit=Hardware notes: A delay line is a chain, each link of which is an SRL16 followed by a flip-flop. If register retiming is enabled, the delay line is a chain of flip-flops.  xl_area=[2 4 0 0 0 0 0]  block_version=VER_STRING_GOES_HERE
  Delay1 : xbsIndex_r4/Delay  en=off  latency=1  dbl_ovrd=off  reg_retiming=off  block_type=delay
      settings: infoedit=Hardware notes: A delay line is a chain, each link of which is an SRL16 followed by a flip-flop. If register retiming is enabled, the delay line is a chain of flip-flops.  xl_area=[2 4 0 0 0 0 0]  block_version=VER_STRING_GOES_HERE
  Mux2 : xbsIndex_r4/Mux  inputs=9  en=off  latency=0  precision=User Defined  arith_type=Unsigned  n_bits=8  bin_pt=0  quantization=Truncate  overflow=Wrap  dbl_ovrd=off  block_type=mux
      settings: xl_area=[36 0 0 72 0 0 0]  block_version=VER_STRING_GOES_HERE
  ROM : xbsIndex_r4/ROM  depth=4  initVector=[0 1 3 2]  distributed_mem=Distributed memory  rst=off  init_reg=0  en=off  latency=1  arith_type=Unsigned  n_bits=2  bin_pt=0  dbl_ovrd=off  optimize=Area  use_rpm=off  block_type=sprom
      settings: xl_area=[1 2 0 2 0 0 0]  block_version=VER_STRING_GOES_HERE
  ROM1 : xbsIndex_r4/ROM  depth=4  initVector=[0 1 3 2]  distributed_mem=Distributed memory  rst=off  init_reg=0  en=off  latency=1  arith_type=Unsigned  n_bits=2  bin_pt=0  dbl_ovrd=off  optimize=Area  use_rpm=off  block_type=sprom
      settings: xl_area=[1 2 0 2 0 0 0]  block_version=VER_STRING_GOES_HERE
  ROM2 : xbsIndex_r4/ROM  depth=8  initVector=[1 0 2 3 7 6 4 5]  distributed_mem=Distributed memory  rst=off  init_reg=0  en=off  latency=1  arith_type=Unsigned  n_bits=3  bin_pt=0  dbl_ovrd=off  optimize=Area  use_rpm=off  block_type=sprom
      settings: xl_area=[2 3 0 3 0 0 0]  block_version=VER_STRING_GOES_HERE
  ROM3 : xbsIndex_r4/ROM  depth=8  initVector=[1 0 2 3 7 6 4 5]  distributed_mem=Distributed memory  rst=off  init_reg=0  en=off  latency=1  arith_type=Unsigned  n_bits=3  bin_pt=0  dbl_ovrd=off  optimize=Area  use_rpm=off  block_type=sprom
      settings: xl_area=[2 3 0 3 0 0 0]  block_version=VER_STRING_GOES_HERE
  ROM4 : xbsIndex_r4/ROM  depth=16  initVector=[1 0 2 3 5 4 6 7 15 14 12 13 11 10 8 9]  distributed_mem=Distributed memory  rst=off  init_reg=0  en=off  latency=1  arith_type=Unsigned  n_bits=4  bin_pt=0  dbl_ovrd=off  optimize=Area  use_rpm=off  block_type=sprom
      settings: xl_area=[2 4 0 4 0 0 0]  block_version=VER_STRING_GOES_HERE
  ROM5 : xbsIndex_r4/ROM  depth=16  initVector=[1 0 2 3 5 4 6 7 15 14 12 13 11 10 8 9]  distributed_mem=Distributed memory  rst=off  init_reg=0  en=off  latency=1  arith_type=Unsigned  n_bits=4  bin_pt=0  dbl_ovrd=off  optimize=Area  use_rpm=off  block_type=sprom
      settings: xl_area=[2 4 0 4 0 0 0]  block_version=VER_STRING_GOES_HERE
  Slice : xbsIndex_r4/Slice  nbits=1  boolean_output=off  mode=Upper Bit Location + Width  bit1=0  base1=MSB of Input  bit0=0  base0=LSB of Input  dbl_ovrd=off  block_type=slice
      settings: infoedit="Extracts a given range of bits from each input sample and presents it at the output.  The output type is ordinarily unsigned with binary point at zero, but can be Boolean when the slice is one bit wide.<P><P>Hardware notes: In hardware this block costs nothing."  block_version=VER_STRING_GOES_HERE
  Slice1 : xbsIndex_r4/Slice  nbits=1  boolean_output=off  mode=Upper Bit Location + Width  bit1=0  base1=MSB of Input  bit0=0  base0=LSB of Input  dbl_ovrd=off  block_type=slice
      settings: infoedit="Extracts a given range of bits from each input sample and presents it at the output.  The output type is ordinarily unsigned with binary point at zero, but can be Boolean when the slice is one bit wide.<P><P>Hardware notes: In hardware this block costs nothing."  block_version=VER_STRING_GOES_HERE
  Slice2 : xbsIndex_r4/Slice  nbits=2  boolean_output=off  mode=Upper Bit Location + Width  bit1=0  base1=MSB of Input  bit0=0  base0=LSB of Input  dbl_ovrd=off  block_type=slice
      settings: infoedit="Extracts a given range of bits from each input sample and presents it at the output.  The output type is ordinarily unsigned with binary point at zero, but can be Boolean when the slice is one bit wide.<P><P>Hardware notes: In hardware this block costs nothing."  block_version=VER_STRING_GOES_HERE
  Slice3 : xbsIndex_r4/Slice  nbits=2  boolean_output=off  mode=Upper Bit Location + Width  bit1=0  base1=MSB of Input  bit0=0  base0=LSB of Input  dbl_ovrd=off  block_type=slice
      settings: infoedit="Extracts a given range of bits from each input sample and presents it at the output.  The output type is ordinarily unsigned with binary point at zero, but can be Boolean when the slice is one bit wide.<P><P>Hardware notes: In hardware this block costs nothing."  block_version=VER_STRING_GOES_HERE
  Slice4 : xbsIndex_r4/Slice  nbits=3  boolean_output=off  mode=Upper Bit Location + Width  bit1=0  base1=MSB of Input  bit0=0  base0=LSB of Input  dbl_ovrd=off  block_type=slice
      settings: infoedit="Extracts a given range of bits from each input sample and presents it at the output.  The output type is ordinarily unsigned with binary point at zero, but can be Boolean when the slice is one bit wide.<P><P>Hardware notes: In hardware this block costs nothing."  block_version=VER_STRING_GOES_HERE
  Slice5 : xbsIndex_r4/Slice  nbits=3  boolean_output=off  mode=Upper Bit Location + Width  bit1=0  base1=MSB of Input  bit0=0  base0=LSB of Input  dbl_ovrd=off  block_type=slice
      settings: infoedit="Extracts a given range of bits from each input sample and presents it at the output.  The output type is ordinarily unsigned with binary point at zero, but can be Boolean when the slice is one bit wide.<P><P>Hardware notes: In hardware this block costs nothing."  block_version=VER_STRING_GOES_HERE
  Slice{6..9} (4 alike) : xbsIndex_r4/Slice  nbits=4  boolean_output=off  mode=Upper Bit Location + Width  bit1=0  base1=MSB of Input  bit0=0  base0=LSB of Input  dbl_ovrd=off  block_type=slice
      settings: infoedit="Extracts a given range of bits from each input sample and presents it at the output.  The output type is ordinarily unsigned with binary point at zero, but can be Boolean when the slice is one bit wide.<P><P>Hardware notes: In hardware this block costs nothing."  block_version=VER_STRING_GOES_HERE
  bits : Outport  OutDataType=sfix(16)  OutScaling=2^0
  wires:
  Delay/1 -> Concat/1, Mux2/3
  Delay1/1 -> Concat/2
  Slice9/1 -> Slice/1, Slice4/1, Slice6/1, Slice2/1
  Slice8/1 -> Slice5/1, Slice7/1, Slice3/1, Slice1/1
  Slice5/1 -> ROM3/1
  Slice4/1 -> ROM2/1
  ROM3/1 -> Concat6/2
  ROM2/1 -> Concat6/1
  ROM1/1 -> Concat5/2
  ROM/1 -> Concat5/1
  ROM5/1 -> Concat1/2
  ROM4/1 -> Concat1/1
  Slice7/1 -> ROM5/1
  Slice6/1 -> ROM4/1
  Concat1/1 -> Mux2/10
  Constant7/1 -> Mux2/7, Mux2/5, Mux2/2, Mux2/9
  Mux2/1 -> bits/1
  Mod Sel/1 -> Mux2/1
  Concat6/1 -> Mux2/8
  Concat5/1 -> Mux2/6
  Slice3/1 -> ROM1/1
  Slice2/1 -> ROM/1
  Concat/1 -> Mux2/4
  Q/1 -> Slice8/1
  Slice1/1 -> Delay1/1
  Slice/1 -> Delay/1
  I/1 -> Slice9/1

== system ofdm_txrx_supermimo/OFDM Rx MIMO/Equalizer & Packetizer/Packet_Constructor/Modulation RAM ==
  Xk_index : Inport  OutDataType=sfix(16)  OutScaling=2^0
  Sym Index : Inport  Port=2  OutDataType=sfix(16)  OutScaling=2^0
  FullRateMasks : Inport  Port=3  OutDataType=sfix(16)  OutScaling=2^0
  1MSB-1 : xbsIndex_r4/Slice  nbits=1  boolean_output=off  mode=Upper Bit Location + Width  bit1=-1  base1=MSB of Input  bit0=0  base0=LSB of Input  dbl_ovrd=off  block_type=slice
      settings: infoedit="Extracts a given range of bits from each input sample and presents it at the output.  The output type is ordinarily unsigned with binary point at zero, but can be Boolean when the slice is one bit wide.<P><P>Hardware notes: In hardware this block costs nothing."  block_version=VER_STRING_GOES_HERE
  4LSB : xbsIndex_r4/Slice  nbits=4  boolean_output=off  mode=Lower Bit Location + Width  bit1=0  base1=MSB of Input  bit0=0  base0=LSB of Input  dbl_ovrd=off  block_type=slice
      settings: infoedit="Extracts a given range of bits from each input sample and presents it at the output.  The output type is ordinarily unsigned with binary point at zero, but can be Boolean when the slice is one bit wide.<P><P>Hardware notes: In hardware this block costs nothing."  block_version=VER_STRING_GOES_HERE
  4MSB : xbsIndex_r4/Slice  nbits=4  boolean_output=off  mode=Upper Bit Location + Width  bit1=0  base1=MSB of Input  bit0=0  base0=LSB of Input  dbl_ovrd=off  block_type=slice
      settings: infoedit="Extracts a given range of bits from each input sample and presents it at the output.  The output type is ordinarily unsigned with binary point at zero, but can be Boolean when the slice is one bit wide.<P><P>Hardware notes: In hardware this block costs nothing."  block_version=VER_STRING_GOES_HERE
  Addr Gen : Subsystem
  Concat : xbsIndex_r4/Concat  num_inputs=4  dbl_ovrd=off  block_type=concat
      settings: block_version=VER_STRING_GOES_HERE
  Concat1 : xbsIndex_r4/Concat  num_inputs=4  dbl_ovrd=off  block_type=concat
      settings: block_version=VER_STRING_GOES_HERE
  Concat2 : xbsIndex_r4/Concat  num_inputs=4  dbl_ovrd=off  block_type=concat
      settings: block_version=VER_STRING_GOES_HERE
  Delay : xbsIndex_r4/Delay  en=off  latency=1  dbl_ovrd=off  reg_retiming=off  block_type=delay
      settings: infoedit=Hardware notes: A delay line is a chain, each link of which is an SRL16 followed by a flip-flop. If register retiming is enabled, the delay line is a chain of flip-flops.  block_version=8.2.02
  Delay1 : xbsIndex_r4/Delay  en=off  latency=1  dbl_ovrd=off  reg_retiming=off  block_type=delay
      settings: infoedit=Hardware notes: A delay line is a chain, each link of which is an SRL16 followed by a flip-flop. If register retiming is enabled, the delay line is a chain of flip-flops.  block_version=8.2.02
  Inverter : xbsIndex_r4/Inverter  en=off  latency=1  dbl_ovrd=off  block_type=inv
      settings: block_version=VER_STRING_GOES_HERE
  Inverter1 : xbsIndex_r4/Inverter  en=off  latency=0  dbl_ovrd=off  block_type=inv
      settings: block_version=VER_STRING_GOES_HERE
  Inverter2 : xbsIndex_r4/Inverter  en=off  latency=0  dbl_ovrd=off  block_type=inv
      settings: block_version=VER_STRING_GOES_HERE
  Logical : xbsIndex_r4/Logical  logical_function=AND  inputs=2  en=off  latency=0  precision=Full  arith_type=Unsigned  n_bits=16  bin_pt=0  align_bp=on  dbl_ovrd=off  block_type=logical
      settings: block_version=VER_STRING_GOES_HERE
  Logical2 : xbsIndex_r4/Logical  logical_function=AND  inputs=2  en=off  latency=0  precision=Full  arith_type=Unsigned  n_bits=16  bin_pt=0  align_bp=on  dbl_ovrd=off  block_type=logical
      settings: block_version=VER_STRING_GOES_HERE
  Logical3 : xbsIndex_r4/Logical  logical_function=OR  inputs=3  en=off  latency=0  precision=Full  arith_type=Unsigned  n_bits=16  bin_pt=0  align_bp=on  dbl_ovrd=off  block_type=logical
      settings: block_version=VER_STRING_GOES_HERE
  Modulation Masks : Subsystem
  Mux1 : xbsIndex_r4/Mux  inputs=2  en=off  latency=0  precision=Full  arith_type=Unsigned  n_bits=16  bin_pt=14  quantization=Truncate  overflow=Wrap  dbl_ovrd=off  block_type=mux
      settings: block_version=8.2.02
  mod : Outport  OutDataType=sfix(16)  OutScaling=2^0
  wires:
  Xk_index/1 -> Addr Gen/1(Xk_index)
  Sym Index/1 -> Addr Gen/2(Sym Index)
  Addr Gen/1(IgnoreSym) -> Inverter/1
  Inverter/1 -> Concat/4, Concat/3, Concat/2, Concat/1
  Addr Gen/2(Mod Addr) -> 1MSB-1/1, Modulation Masks/1(Addr)
  Logical/1 -> mod/1
  Addr Gen/3(FullRate) -> Delay/1
  1MSB-1/1 -> Delay1/1
  FullRateMasks/1 -> 4LSB/1, 4MSB/1
  4LSB/1 -> Mux1/2
  4MSB/1 -> Mux1/3
  Mux1/1 -> Logical3/2
  Logical2/1 -> Logical/1
  Logical3/1 -> Logical2/1
  Concat/1 -> Logical2/2
  Concat1/1 -> Logical3/1
  [regRx_simpleDynRxModulationEn] -> Inverter1/1
  Inverter1/1 -> Concat1/1, Concat1/2, Concat1/3, Concat1/4
  Concat2/1 -> Logical3/3
  Inverter2/1 -> Concat2/1, Concat2/4, Concat2/3, Concat2/2
  Delay/1 -> Inverter2/1
  Delay1/1 -> Mux1/1
  Modulation Masks/1(ModMask) -> Logical/2

== system ofdm_txrx_supermimo/OFDM Rx MIMO/Equalizer & Packetizer/Packet_Constructor/Slicer ==
  note: 8MSB
  note: 8MSB-7
  SliceSel : Inport  OutDataType=sfix(16)  OutScaling=2^0
  D : Inport  Port=2  OutDataType=sfix(16)  OutScaling=2^0
  " byte mux1" : xbsIndex_r4/Mux  inputs=8  en=off  latency=0  precision=Full  arith_type="Signed  (2's comp)"  n_bits=8  bin_pt=2  quantization=Truncate  overflow=Wrap  dbl_ovrd=off  block_type=mux
      settings: xl_area=[16 0 0 32 0 0 0]  block_version=VER_STRING_GOES_HERE
  Slice : xbsIndex_r4/Slice  nbits=8  boolean_output=off  mode=Lower Bit Location + Width  bit1=0  base1=MSB of Input  bit0=0  base0=LSB of Input  dbl_ovrd=off  block_type=slice
      settings: infoedit="Extracts a given range of bits from each input sample and presents it at the output.  The output type is ordinarily unsigned with binary point at zero, but can be Boolean when the slice is one bit wide.<P><P>Hardware notes: In hardware this block costs nothing."  block_version=VER_STRING_GOES_HERE
  Slice1 : xbsIndex_r4/Slice  nbits=8  boolean_output=off  mode=Lower Bit Location + Width  bit1=0  base1=MSB of Input  bit0=1  base0=LSB of Input  dbl_ovrd=off  block_type=slice
      settings: infoedit="Extracts a given range of bits from each input sample and presents it at the output.  The output type is ordinarily unsigned with binary point at zero, but can be Boolean when the slice is one bit wide.<P><P>Hardware notes: In hardware this block costs nothing."  block_version=VER_STRING_GOES_HERE
  Slice2 : xbsIndex_r4/Slice  nbits=8  boolean_output=off  mode=Lower Bit Location + Width  bit1=0  base1=MSB of Input  bit0=2  base0=LSB of Input  dbl_ovrd=off  block_type=slice
      settings: infoedit="Extracts a given range of bits from each input sample and presents it at the output.  The output type is ordinarily unsigned with binary point at zero, but can be Boolean when the slice is one bit wide.<P><P>Hardware notes: In hardware this block costs nothing."  block_version=VER_STRING_GOES_HERE
  Slice3 : xbsIndex_r4/Slice  nbits=8  boolean_output=off  mode=Lower Bit Location + Width  bit1=0  base1=MSB of Input  bit0=3  base0=LSB of Input  dbl_ovrd=off  block_type=slice
      settings: infoedit="Extracts a given range of bits from each input sample and presents it at the output.  The output type is ordinarily unsigned with binary point at zero, but can be Boolean when the slice is one bit wide.<P><P>Hardware notes: In hardware this block costs nothing."  block_version=VER_STRING_GOES_HERE
  Slice4 : xbsIndex_r4/Slice  nbits=8  boolean_output=off  mode=Lower Bit Location + Width  bit1=0  base1=MSB of Input  bit0=4  base0=LSB of Input  dbl_ovrd=off  block_type=slice
      settings: infoedit="Extracts a given range of bits from each input sample and presents it at the output.  The output type is ordinarily unsigned with binary point at zero, but can be Boolean when the slice is one bit wide.<P><P>Hardware notes: In hardware this block costs nothing."  block_version=VER_STRING_GOES_HERE
  Slice5 : xbsIndex_r4/Slice  nbits=8  boolean_output=off  mode=Lower Bit Location + Width  bit1=0  base1=MSB of Input  bit0=5  base0=LSB of Input  dbl_ovrd=off  block_type=slice
      settings: infoedit="Extracts a given range of bits from each input sample and presents it at the output.  The output type is ordinarily unsigned with binary point at zero, but can be Boolean when the slice is one bit wide.<P><P>Hardware notes: In hardware this block costs nothing."  block_version=VER_STRING_GOES_HERE
  Slice6 : xbsIndex_r4/Slice  nbits=8  boolean_output=off  mode=Lower Bit Location + Width  bit1=0  base1=MSB of Input  bit0=6  base0=LSB of Input  dbl_ovrd=off  block_type=slice
      settings: infoedit="Extracts a given range of bits from each input sample and presents it at the output.  The output type is ordinarily unsigned with binary point at zero, but can be Boolean when the slice is one bit wide.<P><P>Hardware notes: In hardware this block costs nothing."  block_version=VER_STRING_GOES_HERE
  Slice7 : xbsIndex_r4/Slice  nbits=8  boolean_output=off  mode=Lower Bit Location + Width  bit1=0  base1=MSB of Input  bit0=7  base0=LSB of Input  dbl_ovrd=off  block_type=slice
      settings: infoedit="Extracts a given range of bits from each input sample and presents it at the output.  The output type is ordinarily unsigned with binary point at zero, but can be Boolean when the slice is one bit wide.<P><P>Hardware notes: In hardware this block costs nothing."  block_version=VER_STRING_GOES_HERE
  Q : Outport  OutDataType=sfix(16)  OutScaling=2^0
  wires:
  " byte mux1"/1 -> Q/1
  SliceSel/1 -> " byte mux1"/1
  D/1 -> Slice3/1, Slice6/1, Slice7/1, Slice5/1, Slice4/1, Slice2/1, Slice1/1, Slice/1
  Slice/1 -> " byte mux1"/2
  Slice1/1 -> " byte mux1"/3
  Slice2/1 -> " byte mux1"/4
  Slice3/1 -> " byte mux1"/5
  Slice4/1 -> " byte mux1"/6
  Slice5/1 -> " byte mux1"/7
  Slice6/1 -> " byte mux1"/8
  Slice7/1 -> " byte mux1"/9

== system ofdm_txrx_supermimo/OFDM Rx MIMO/FFT & Chan Est/Addr Gen1/Copy_Downsample ==
  D : Inport  OutDataType=sfix(16)  OutScaling=2^0
  Delay : xbsIndex_r4/Delay  en=off  latency=1  dbl_ovrd=off  reg_retiming=off  block_type=delay
      settings: infoedit=Hardware notes: A delay line is a chain, each link of which is an SRL16 followed by a flip-flop. If register retiming is enabled, the delay line is a chain of flip-flops.  block_version=VER_STRING_GOES_HERE
  Down Sample : xbsIndex_r4/Down Sample  sample_ratio=2  sample_phase="Last Value of Frame  (most efficient)"  en=off  latency=1  dbl_ovrd=off  block_type=dsamp
      settings: block_version=VER_STRING_GOES_HERE
  Logical : xbsIndex_r4/Logical  logical_function=OR  inputs=2  en=off  latency=0  precision=Full  arith_type=Unsigned  n_bits=16  bin_pt=0  align_bp=on  dbl_ovrd=off  block_type=logical
      settings: block_version=VER_STRING_GOES_HERE
  Q : Outport  OutDataType=sfix(16)  OutScaling=2^0
  wires:
  Delay/1 -> Logical/1
  D/1 -> Delay/1, Logical/2
  Logical/1 -> Down Sample/1
  Down Sample/1 -> Q/1
